simulink model "AcasXuClosedLoop_all_comp"  (Simulink 10.0)
settings: Solver=VariableStepAuto  StartTime=0.0  StopTime=20  RelTol=1e-3
libraries: neural x14040, simulink x2
(part 29 of 534)

== system AcasXuClosedLoop_all_comp/networks/Networks_m_7/AcasXu_1_7/Process Input 1 ==
  x : Inport  PortDimensions=5
      mask Display: e = 0.06;c1 = [120 230 180]/255;c2 = ([120 230 180]+0)/(256+128);c3 = ([120 230 180]+128)/(256+128);patch([0 1 1 0],[0 0 1 1],c1);patch([0 1 1 1-e 1-e 0+e],[0 0 1 1-e 0+e 0+e],c2);patch([0 0 1 1-e 0+e 0+e],[0 1 1 1-e 1-e 0+e],c3);plot([0 1 1 0 0],[0 0 1 1 0]);
  p : Outport  PortDimensions=5
      mask Display: e = 0.06;c1 = [120 230 180]/255;c2 = ([120 230 180]+0)/(256+128);c3 = ([120 230 180]+128)/(256+128);patch([0 1 1 0],[0 0 1 1],c1);patch([0 1 1 1-e 1-e 0+e],[0 0 1 1-e 0+e 0+e],c2);patch([0 0 1 1-e 0+e 0+e],[0 1 1 1-e 1-e 0+e],c3);plot([0 1 1 0 0],[0 0 1 1 0]);
  wires:
  x/1 -> p/1

== system AcasXuClosedLoop_all_comp/networks/Networks_m_7/AcasXu_1_7/Process Output 1 ==
  a : Inport  PortDimensions=5
      mask Display: e = 0.06;c1 = [120 230 180]/255;c2 = ([120 230 180]+0)/(256+128);c3 = ([120 230 180]+128)/(256+128);patch([0 1 1 0],[0 0 1 1],c1);patch([0 1 1 1-e 1-e 0+e],[0 0 1 1-e 0+e 0+e],c2);patch([0 0 1 1-e 0+e 0+e],[0 1 1 1-e 1-e 0+e],c3);plot([0 1 1 0 0],[0 0 1 1 0]);
  y : Outport  PortDimensions=5
      mask Display: e = 0.06;c1 = [120 230 180]/255;c2 = ([120 230 180]+0)/(256+128);c3 = ([120 230 180]+128)/(256+128);patch([0 1 1 0],[0 0 1 1],c1);patch([0 1 1 1-e 1-e 0+e],[0 0 1 1-e 0+e 0+e],c2);patch([0 0 1 1-e 0+e 0+e],[0 1 1 1-e 1-e 0+e],c3);plot([0 1 1 0 0],[0 0 1 1 0]);
  wires:
  a/1 -> y/1

== system AcasXuClosedLoop_all_comp/networks/Networks_m_7/AcasXu_2_7/Layer 1 ==
  "p{1}" : Inport  PortDimensions=5
      mask Display: e = 0.06;c1 = [120 230 180]/255;c2 = ([120 230 180]+0)/(256+128);c3 = ([120 230 180]+128)/(256+128);patch([0 1 1 0],[0 0 1 1],c1);patch([0 1 1 1-e 1-e 0+e],[0 0 1 1-e 0+e 0+e],c2);patch([0 0 1 1-e 0+e 0+e],[0 1 1 1-e 1-e 0+e],c3);plot([0 1 1 0 0],[0 0 1 1 0]);
  Delays 1 : Subsystem
      mask Display: e = 0.06;c1 = [120 150 230]/255;c2 = ([120 150 230]+0)/383;c3 = ([120 150 230]+128)/383;patch([0 1 1 0],[0 0 1 1],c1);patch([0 1 1 1-e 1-e 0+e],[0 0 1 1-e 0+e 0+e],c2);patch([0 0 1 1-e 0+e 0+e],[0 1 1 1-e 1-e 0+e],c3);plot([0 1 1 0 0],[0 0 1 1 0]);disp('0');
  "IW{1,1}" : Subsystem
      mask Display: e = 0.06;c1 = [120 150 230]/255;c2 = ([120 150 230]+0)/383;c3 = ([120 150 230]+128)/383;patch([0 1 1 0],[0 0 1 1],c1);patch([0 1 1 1-e 1-e 0+e],[0 0 1 1-e 0+e 0+e],c2);patch([0 0 1 1-e 0+e 0+e],[0 1 1 1-e 1-e 0+e],c3);plot([0 1 1 0 0],[0 0 1 1 0]);disp('W');
  "b{1}" : Constant  Value=[0.5234229999999999716209231337415985763072967529296875;0.11526999999999999746425061175614246167242527008056640625;-0.10370600000000000651123599482161807827651500701904296875;0.07139189999999999425295271748836967162787914276123046875;-0.1364549999999999929656269159750081598758697509765625;-0.48813600000000001433164698028122074902057647705078125;-0.257603000000000026403768060845322906970977783203125;-0.18697500000000000230926389122032560408115386962890625;-0.18462400000000001032418595059425570070743560791015625;0.068212400000000006361489113260176964104175567626953125;-0.2209480000000000055049298453013761900365352630615234375;-0.025648600000000000564970292771249660290777683258056640625;0.09991239999999999843449671743655926547944545745849609375;-0.0200602999999999996594279849659869796596467494964599609375;-0.5281029999999999891002744334400631487369537353515625;-0.01088579999999999929627403361109827528707683086395263671875;0.0243305000000000014315215679516768432222306728363037109375;-0.33044499999999998873789763820241205394268035888671875;0.12378599999999999325961397289574961178004741668701171875;0.0128616000000000008096634473986341618001461029052734375;-0.414652999999999993807620057850726880133152008056640625;-0.56115300000000001290345608140341937541961669921875;-0.007621389999999999588509158598981230170466005802154541015625;-0.751859000000000055052851166692562401294708251953125;-0.2264529999999999876347800409348565153777599334716796875;-0.003468859999999999825515129003861147793941199779510498046875;-0.32769700000000001605116040082066319882869720458984375;0.072733199999999997853450395268737338483333587646484375;-0.51464699999999996560262616185355000197887420654296875;0.01305259999999999927233762520017990027554333209991455078125;-0.5564270000000000049311665861750952899456024169921875;-0.29400199999999998556887703671236522495746612548828125;-0.457662999999999986489029879521694965660572052001953125;-0.1156689999999999940438755174909601919353008270263671875;-0.2459050000000000124611432283927570097148418426513671875;0.54282799999999997719868360945838503539562225341796875;-0.318711000000000022058799231672310270369052886962890625;-0.6888609999999999455866372954915277659893035888671875;-0.1474320000000000074891204349114559590816497802734375;-0.366167999999999993487875826758681796491146087646484375;0.1375860000000000138431488494461518712341785430908203125;-0.8581119999999999858886212678044103085994720458984375;0.0916486999999999996102673094355850480496883392333984375;0.08870020000000000681694700688240118324756622314453125;0.05061659999999999770547987054669647477567195892333984375;-0.376475999999999977330134015574003569781780242919921875;0.0620319000000000009276135415348107926547527313232421875;0.31844200000000000283506551568279974162578582763671875;-0.2102920000000000066986416413783445023000240325927734375;0.318415999999999976832754100541933439671993255615234375]
      mask Display: e = 0.06;c1 = [120 150 230]/255;c2 = ([120 150 230]+0)/383;c3 = ([120 150 230]+128)/383;patch([0 1 1 0],[0 0 1 1],c1);patch([0 1 1 1-e 1-e 0+e],[0 0 1 1-e 0+e 0+e],c2);patch([0 0 1 1-e 0+e 0+e],[0 1 1 1-e 1-e 0+e],c3);plot([0 1 1 0 0],[0 0 1 1 0]);disp('b');
  netsum : Sum  Inputs=2  SaturateOnIntegerOverflow=on  InputSameDT=on  OutDataTypeStr=Inherit: Same as first input
      mask Display: e = 0.06;c1 = [120 150 230]/255;c2 = ([120 150 230]+0)/383;c3 = ([120 150 230]+128)/383;patch([0 1 1 0],[0 0 1 1],c1);patch([0 1 1 1-e 1-e 0+e],[0 0 1 1-e 0+e 0+e],c2);patch([0 0 1 1-e 0+e 0+e],[0 1 1 1-e 1-e 0+e],c3);plot([0 1 1 0 0],[0 0 1 1 0]);disp('+');
  poslin : neural/Transfer Functions/poslin
      settings: SourceProductName=Deep Learning Toolbox  SourceProductBaseCode=NN
  "a{1}" : Outport  InitialOutput=[0;0;0;0;0;0;0;0;0;0;0;0;0;0;0;0;0;0;0;0;0;0;0;0;0;0;0;0;0;0;0;0;0;0;0;0;0;0;0;0;0;0;0;0;0;0;0;0;0;0]
      mask Display: e = 0.06;c1 = [120 230 180]/255;c2 = ([120 230 180]+0)/(256+128);c3 = ([120 230 180]+128)/(256+128);patch([0 1 1 0],[0 0 1 1],c1);patch([0 1 1 1-e 1-e 0+e],[0 0 1 1-e 0+e 0+e],c2);patch([0 0 1 1-e 0+e 0+e],[0 1 1 1-e 1-e 0+e],c3);plot([0 1 1 0 0],[0 0 1 1 0]);
  wires:
  poslin/1 -> "a{1}"/1
  netsum/1 -> poslin/1
  "b{1}"/1 -> netsum/2
  "IW{1,1}"/1("iz{1,1}") -> netsum/1
  Delays 1/1("pd{1,1}") -> "IW{1,1}"/1("pd{1,1}")
  "p{1}"/1 -> Delays 1/1("p{1}")

== system AcasXuClosedLoop_all_comp/networks/Networks_m_7/AcasXu_2_7/Layer 2 ==
  "a{1} " : Inport  PortDimensions=50
      mask Display: e = 0.06;c1 = [120 230 180]/255;c2 = ([120 230 180]+0)/(256+128);c3 = ([120 230 180]+128)/(256+128);patch([0 1 1 0],[0 0 1 1],c1);patch([0 1 1 1-e 1-e 0+e],[0 0 1 1-e 0+e 0+e],c2);patch([0 0 1 1-e 0+e 0+e],[0 1 1 1-e 1-e 0+e],c3);plot([0 1 1 0 0],[0 0 1 1 0]);
  Delays 1 : Subsystem
      mask Display: e = 0.06;c1 = [120 150 230]/255;c2 = ([120 150 230]+0)/383;c3 = ([120 150 230]+128)/383;patch([0 1 1 0],[0 0 1 1],c1);patch([0 1 1 1-e 1-e 0+e],[0 0 1 1-e 0+e 0+e],c2);patch([0 0 1 1-e 0+e 0+e],[0 1 1 1-e 1-e 0+e],c3);plot([0 1 1 0 0],[0 0 1 1 0]);disp('0');
  "LW{2,1}" : Subsystem
      mask Display: e = 0.06;c1 = [120 150 230]/255;c2 = ([120 150 230]+0)/383;c3 = ([120 150 230]+128)/383;patch([0 1 1 0],[0 0 1 1],c1);patch([0 1 1 1-e 1-e 0+e],[0 0 1 1-e 0+e 0+e],c2);patch([0 0 1 1-e 0+e 0+e],[0 1 1 1-e 1-e 0+e],c3);plot([0 1 1 0 0],[0 0 1 1 0]);disp('W');
  "b{2}" : Constant  Value=[-0.304109000000000018193446749137365259230136871337890625;-0.8032500000000000195399252334027551114559173583984375;0.790129999999999999005240169935859739780426025390625;-0.92732599999999998363620079544489271938800811767578125;-1.424350000000000004973799150320701301097869873046875;-0.0396675999999999973066877601013402454555034637451171875;-0.015490600000000000202593497533598565496504306793212890625;-0.25707000000000002071232074740692041814327239990234375;-0.63598900000000002652456032592453993856906890869140625;0.2584779999999999855475607546395622193813323974609375;-1.148800000000000043343106881366111338138580322265625;0.802705000000000001847411112976260483264923095703125;-0.306186000000000013709922086491133086383342742919921875;-1.356139999999999901092451182194054126739501953125;0.231009999999999993125499031521030701696872711181640625;-0.3563169999999999948414597383816726505756378173828125;-0.0256099000000000014132250925058542634360492229461669921875;-1.553020000000000067075234255753457546234130859375;-0.016525800000000000211652917414539842866361141204833984375;0.03723400000000000320543591669775196351110935211181640625;1.204029999999999933635308480006642639636993408203125;0.884560000000000012931877790833823382854461669921875;0.7280889999999999862012600715388543903827667236328125;-0.028294199999999998518251942414281074889004230499267578125;-1.2106399999999999383959448095993138849735260009765625;0.61121700000000001029576424116385169327259063720703125;-0.01231570000000000060513816180218782392330467700958251953125;0.130025000000000001687538997430237941443920135498046875;-0.00610049000000000007926104217403917573392391204833984375;0.78768800000000005478995035446132533252239227294921875;0.9423880000000000034532376957940869033336639404296875;-0.0214050999999999998213429108773198095150291919708251953125;-0.92050200000000004241229589752038009464740753173828125;-0.0735737999999999947586815096656209789216518402099609375;0.03393319999999999669881844965857453644275665283203125;0.382917000000000007364775456153438426554203033447265625;-1.018219999999999902939862295170314610004425048828125;-0.015413400000000000711342096337830298580229282379150390625;0.5833850000000000424194013248779810965061187744140625;-0.0159619000000000010874856570808333344757556915283203125;0.58658299999999996554578274299274198710918426513671875;0.65938499999999999889865875957184471189975738525390625;0.59477700000000000013500311979441903531551361083984375;1.089960000000000039932501749717630445957183837890625;-0.0159193000000000008109513061071993433870375156402587890625;-0.4555919999999999969730879456619732081890106201171875;-0.109399999999999997246646898929611779749393463134765625;0.57275500000000001410427330483798868954181671142578125;-0.0150588000000000006572964394990776781924068927764892578125;-0.0170725000000000008693046282814975711517035961151123046875]
      mask Display: e = 0.06;c1 = [120 150 230]/255;c2 = ([120 150 230]+0)/383;c3 = ([120 150 230]+128)/383;patch([0 1 1 0],[0 0 1 1],c1);patch([0 1 1 1-e 1-e 0+e],[0 0 1 1-e 0+e 0+e],c2);patch([0 0 1 1-e 0+e 0+e],[0 1 1 1-e 1-e 0+e],c3);plot([0 1 1 0 0],[0 0 1 1 0]);disp('b');
  netsum : Sum  Inputs=2  SaturateOnIntegerOverflow=on  InputSameDT=on  OutDataTypeStr=Inherit: Same as first input
      mask Display: e = 0.06;c1 = [120 150 230]/255;c2 = ([120 150 230]+0)/383;c3 = ([120 150 230]+128)/383;patch([0 1 1 0],[0 0 1 1],c1);patch([0 1 1 1-e 1-e 0+e],[0 0 1 1-e 0+e 0+e],c2);patch([0 0 1 1-e 0+e 0+e],[0 1 1 1-e 1-e 0+e],c3);plot([0 1 1 0 0],[0 0 1 1 0]);disp('+');
  poslin : neural/Transfer Functions/poslin
      settings: SourceProductName=Deep Learning Toolbox  SourceProductBaseCode=NN
  "a{2}" : Outport  InitialOutput=[0;0;0;0;0;0;0;0;0;0;0;0;0;0;0;0;0;0;0;0;0;0;0;0;0;0;0;0;0;0;0;0;0;0;0;0;0;0;0;0;0;0;0;0;0;0;0;0;0;0]
      mask Display: e = 0.06;c1 = [120 230 180]/255;c2 = ([120 230 180]+0)/(256+128);c3 = ([120 230 180]+128)/(256+128);patch([0 1 1 0],[0 0 1 1],c1);patch([0 1 1 1-e 1-e 0+e],[0 0 1 1-e 0+e 0+e],c2);patch([0 0 1 1-e 0+e 0+e],[0 1 1 1-e 1-e 0+e],c3);plot([0 1 1 0 0],[0 0 1 1 0]);
  wires:
  poslin/1 -> "a{2}"/1
  netsum/1 -> poslin/1
  "b{2}"/1 -> netsum/2
  "LW{2,1}"/1("lz{2,1}") -> netsum/1
  Delays 1/1("pd{2,1}") -> "LW{2,1}"/1("ad{2,1}")
  "a{1} "/1 -> Delays 1/1("p{2}")

== system AcasXuClosedLoop_all_comp/networks/Networks_m_7/AcasXu_2_7/Layer 3 ==
  "a{2} " : Inport  PortDimensions=50
      mask Display: e = 0.06;c1 = [120 230 180]/255;c2 = ([120 230 180]+0)/(256+128);c3 = ([120 230 180]+128)/(256+128);patch([0 1 1 0],[0 0 1 1],c1);patch([0 1 1 1-e 1-e 0+e],[0 0 1 1-e 0+e 0+e],c2);patch([0 0 1 1-e 0+e 0+e],[0 1 1 1-e 1-e 0+e],c3);plot([0 1 1 0 0],[0 0 1 1 0]);
  Delays 1 : Subsystem
      mask Display: e = 0.06;c1 = [120 150 230]/255;c2 = ([120 150 230]+0)/383;c3 = ([120 150 230]+128)/383;patch([0 1 1 0],[0 0 1 1],c1);patch([0 1 1 1-e 1-e 0+e],[0 0 1 1-e 0+e 0+e],c2);patch([0 0 1 1-e 0+e 0+e],[0 1 1 1-e 1-e 0+e],c3);plot([0 1 1 0 0],[0 0 1 1 0]);disp('0');
  "LW{3,2}" : Subsystem
      mask Display: e = 0.06;c1 = [120 150 230]/255;c2 = ([120 150 230]+0)/383;c3 = ([120 150 230]+128)/383;patch([0 1 1 0],[0 0 1 1],c1);patch([0 1 1 1-e 1-e 0+e],[0 0 1 1-e 0+e 0+e],c2);patch([0 0 1 1-e 0+e 0+e],[0 1 1 1-e 1-e 0+e],c3);plot([0 1 1 0 0],[0 0 1 1 0]);disp('W');
  "b{3}" : Constant  Value=[-0.05460299999999999875921474767892505042254924774169921875;-1.51025000000000009237055564881302416324615478515625;-1.2421100000000000473932004751986823976039886474609375;-1.3791700000000000070343730840249918401241302490234375;-2.25302999999999986613374858279712498188018798828125;-0.06695610000000000450182113809205475263297557830810546875;0.034173500000000002485567307530800462700426578521728515625;0.193807000000000007045031225061393342912197113037109375;-3.100969999999999782147597215953283011913299560546875;-0.327037999999999995370814076522947289049625396728515625;-0.93910800000000005383071766118519008159637451171875;-0.40935500000000002440714297335944138467311859130859375;-1.8680499999999999882760448599583469331264495849609375;0.89845900000000000762412355470587499439716339111328125;-0.33551500000000000767386154620908200740814208984375;-0.1792789999999999939195305387329426594078540802001953125;0.65444899999999994744115383582538925111293792724609375;0.42373899999999997678656882271752692759037017822265625;0.55749300000000001631406121305190026760101318359375;-0.1454959999999999864517263858942897059023380279541015625;0.63791100000000000580513415116001851856708526611328125;0.41257800000000000029132252166164107620716094970703125;0.252634000000000025210056264768354594707489013671875;0.84931199999999995586819068194017745554447174072265625;-0.51852200000000003843325657726381905376911163330078125;0.1806649999999999922639659644119092263281345367431640625;-0.476061000000000011933565247090882621705532073974609375;0.197778000000000009350742402602918446063995361328125;-2.60480000000000000426325641456060111522674560546875;0.3153580000000000271853650701814331114292144775390625;-1.600410000000000110276232589967548847198486328125;0.336000000000000020872192862952942959964275360107421875;-2.201700000000000212452277992269955575466156005859375;0.0999147999999999980591525172712863422930240631103515625;-2.7599900000000001654143488849513232707977294921875;-0.0243119000000000008709921672789278090931475162506103515625;0.65167699999999995075228298446745611727237701416015625;0.319890999999999980918374831162509508430957794189453125;2.00330999999999992411403582082130014896392822265625;-0.9242879999999999984794385454733856022357940673828125;-1.597140000000000004121147867408581078052520751953125;0.5246269999999999544826323472079820930957794189453125;-1.27812999999999998834709913353435695171356201171875;-3.459799999999999986499688020558096468448638916015625;0.37772200000000000219557705349870957434177398681640625;0.95909100000000002683719912965898402035236358642578125;-2.129820000000000046469494918710552155971527099609375;0.08330690000000000317026405127762700431048870086669921875;-0.83285500000000001197264509755768813192844390869140625;-0.5695580000000000087112539404188282787799835205078125]
      mask Display: e = 0.06;c1 = [120 150 230]/255;c2 = ([120 150 230]+0)/383;c3 = ([120 150 230]+128)/383;patch([0 1 1 0],[0 0 1 1],c1);patch([0 1 1 1-e 1-e 0+e],[0 0 1 1-e 0+e 0+e],c2);patch([0 0 1 1-e 0+e 0+e],[0 1 1 1-e 1-e 0+e],c3);plot([0 1 1 0 0],[0 0 1 1 0]);disp('b');
  netsum : Sum  Inputs=2  SaturateOnIntegerOverflow=on  InputSameDT=on  OutDataTypeStr=Inherit: Same as first input
      mask Display: e = 0.06;c1 = [120 150 230]/255;c2 = ([120 150 230]+0)/383;c3 = ([120 150 230]+128)/383;patch([0 1 1 0],[0 0 1 1],c1);patch([0 1 1 1-e 1-e 0+e],[0 0 1 1-e 0+e 0+e],c2);patch([0 0 1 1-e 0+e 0+e],[0 1 1 1-e 1-e 0+e],c3);plot([0 1 1 0 0],[0 0 1 1 0]);disp('+');
  poslin : neural/Transfer Functions/poslin
      settings: SourceProductName=Deep Learning Toolbox  SourceProductBaseCode=NN
  "a{3}" : Outport  InitialOutput=[0;0;0;0;0;0;0;0;0;0;0;0;0;0;0;0;0;0;0;0;0;0;0;0;0;0;0;0;0;0;0;0;0;0;0;0;0;0;0;0;0;0;0;0;0;0;0;0;0;0]
      mask Display: e = 0.06;c1 = [120 230 180]/255;c2 = ([120 230 180]+0)/(256+128);c3 = ([120 230 180]+128)/(256+128);patch([0 1 1 0],[0 0 1 1],c1);patch([0 1 1 1-e 1-e 0+e],[0 0 1 1-e 0+e 0+e],c2);patch([0 0 1 1-e 0+e 0+e],[0 1 1 1-e 1-e 0+e],c3);plot([0 1 1 0 0],[0 0 1 1 0]);
  wires:
  poslin/1 -> "a{3}"/1
  netsum/1 -> poslin/1
  "b{3}"/1 -> netsum/2
  "LW{3,2}"/1("lz{3,2}") -> netsum/1
  Delays 1/1("pd{3,2}") -> "LW{3,2}"/1("ad{3,2}")
  "a{2} "/1 -> Delays 1/1("p{3}")

== system AcasXuClosedLoop_all_comp/networks/Networks_m_7/AcasXu_2_7/Layer 4 ==
  "a{3} " : Inport  PortDimensions=50
      mask Display: e = 0.06;c1 = [120 230 180]/255;c2 = ([120 230 180]+0)/(256+128);c3 = ([120 230 180]+128)/(256+128);patch([0 1 1 0],[0 0 1 1],c1);patch([0 1 1 1-e 1-e 0+e],[0 0 1 1-e 0+e 0+e],c2);patch([0 0 1 1-e 0+e 0+e],[0 1 1 1-e 1-e 0+e],c3);plot([0 1 1 0 0],[0 0 1 1 0]);
  Delays 1 : Subsystem
      mask Display: e = 0.06;c1 = [120 150 230]/255;c2 = ([120 150 230]+0)/383;c3 = ([120 150 230]+128)/383;patch([0 1 1 0],[0 0 1 1],c1);patch([0 1 1 1-e 1-e 0+e],[0 0 1 1-e 0+e 0+e],c2);patch([0 0 1 1-e 0+e 0+e],[0 1 1 1-e 1-e 0+e],c3);plot([0 1 1 0 0],[0 0 1 1 0]);disp('0');
  "LW{4,3}" : Subsystem
      mask Display: e = 0.06;c1 = [120 150 230]/255;c2 = ([120 150 230]+0)/383;c3 = ([120 150 230]+128)/383;patch([0 1 1 0],[0 0 1 1],c1);patch([0 1 1 1-e 1-e 0+e],[0 0 1 1-e 0+e 0+e],c2);patch([0 0 1 1-e 0+e 0+e],[0 1 1 1-e 1-e 0+e],c3);plot([0 1 1 0 0],[0 0 1 1 0]);disp('W');
  "b{4}" : Constant  Value=[-3.171460000000000167830194186535663902759552001953125;0.58932200000000001249844672202016226947307586669921875;-1.4313000000000000166977542903623543679714202880859375;-0.00948605999999999928429250672934358590282499790191650390625;-1.1083700000000000773781039242749102413654327392578125;-1.734809999999999963193886287626810371875762939453125;0.2420529999999999903881331420052447356283664703369140625;0.52557299999999995687716136671951971948146820068359375;-1.92884000000000010999201549566350877285003662109375;2.644420000000000214868123293854296207427978515625;-1.62112999999999995992538970313034951686859130859375;-0.306956999999999979866771582237561233341693878173828125;-4.1185700000000000642330633127130568027496337890625;-1.148260000000000058406612879480235278606414794921875;3.008919999999999816964191268198192119598388671875;2.865229999999999943582906780648045241832733154296875;0.259365999999999985448084771633148193359375;0.4819539999999999935198502498678863048553466796875;0.529708999999999985419663062202744185924530029296875;0.65275499999999997413624441833235323429107666015625;-1.504350000000000076028072726330719888210296630859375;0.90964400000000000812150346973794512450695037841796875;2.1580799999999999982946974341757595539093017578125;3.21356999999999981554310579667799174785614013671875;-0.869021000000000043428372009657323360443115234375;0.75575099999999995059596358260023407638072967529296875;3.0660199999999999675992512493394315242767333984375;-1.489640000000000075175421443418599665164947509765625;0.9238079999999999625259761160123161971569061279296875;1.9984900000000000996891458271420560777187347412109375;0.30948900000000001409006245012278668582439422607421875;-1.6852400000000000712674363967380486428737640380859375;-1.0825400000000000577671244172961451113224029541015625;1.5658000000000000806466005087713710963726043701171875;0.288138999999999978474107820147764869034290313720703125;-0.67373899999999997678656882271752692759037017822265625;-2.964230000000000142534872793476097285747528076171875;-3.2730399999999999494093572138808667659759521484375;0.484302999999999983504750389329274185001850128173828125;0.44874300000000000299138491755002178251743316650390625;-1.372409999999999907771552898338995873928070068359375;1.1985099999999999642597003912669606506824493408203125;-0.0258034000000000006413980457864454365335404872894287109375;-2.59421999999999997044142219237983226776123046875;-0.49597999999999997644550830955267883837223052978515625;-0.75792599999999998860999994576559402048587799072265625;-0.99425799999999997513810967575409449636936187744140625;-1.7488300000000001066524646375910378992557525634765625;-0.1426099999999999867750233306651352904736995697021484375;-1.2576799999999999091215840962831862270832061767578125]
      mask Display: e = 0.06;c1 = [120 150 230]/255;c2 = ([120 150 230]+0)/383;c3 = ([120 150 230]+128)/383;patch([0 1 1 0],[0 0 1 1],c1);patch([0 1 1 1-e 1-e 0+e],[0 0 1 1-e 0+e 0+e],c2);patch([0 0 1 1-e 0+e 0+e],[0 1 1 1-e 1-e 0+e],c3);plot([0 1 1 0 0],[0 0 1 1 0]);disp('b');
  netsum : Sum  Inputs=2  SaturateOnIntegerOverflow=on  InputSameDT=on  OutDataTypeStr=Inherit: Same as first input
      mask Display: e = 0.06;c1 = [120 150 230]/255;c2 = ([120 150 230]+0)/383;c3 = ([120 150 230]+128)/383;patch([0 1 1 0],[0 0 1 1],c1);patch([0 1 1 1-e 1-e 0+e],[0 0 1 1-e 0+e 0+e],c2);patch([0 0 1 1-e 0+e 0+e],[0 1 1 1-e 1-e 0+e],c3);plot([0 1 1 0 0],[0 0 1 1 0]);disp('+');
  poslin : neural/Transfer Functions/poslin
      settings: SourceProductName=Deep Learning Toolbox  SourceProductBaseCode=NN
  "a{4}" : Outport  InitialOutput=[0;0;0;0;0;0;0;0;0;0;0;0;0;0;0;0;0;0;0;0;0;0;0;0;0;0;0;0;0;0;0;0;0;0;0;0;0;0;0;0;0;0;0;0;0;0;0;0;0;0]
      mask Display: e = 0.06;c1 = [120 230 180]/255;c2 = ([120 230 180]+0)/(256+128);c3 = ([120 230 180]+128)/(256+128);patch([0 1 1 0],[0 0 1 1],c1);patch([0 1 1 1-e 1-e 0+e],[0 0 1 1-e 0+e 0+e],c2);patch([0 0 1 1-e 0+e 0+e],[0 1 1 1-e 1-e 0+e],c3);plot([0 1 1 0 0],[0 0 1 1 0]);
  wires:
  poslin/1 -> "a{4}"/1
  netsum/1 -> poslin/1
  "b{4}"/1 -> netsum/2
  "LW{4,3}"/1("lz{4,3}") -> netsum/1
  Delays 1/1("pd{4,3}") -> "LW{4,3}"/1("ad{4,3}")
  "a{3} "/1 -> Delays 1/1("p{4}")

== system AcasXuClosedLoop_all_comp/networks/Networks_m_7/AcasXu_2_7/Layer 5 ==
  "a{4} " : Inport  PortDimensions=50
      mask Display: e = 0.06;c1 = [120 230 180]/255;c2 = ([120 230 180]+0)/(256+128);c3 = ([120 230 180]+128)/(256+128);patch([0 1 1 0],[0 0 1 1],c1);patch([0 1 1 1-e 1-e 0+e],[0 0 1 1-e 0+e 0+e],c2);patch([0 0 1 1-e 0+e 0+e],[0 1 1 1-e 1-e 0+e],c3);plot([0 1 1 0 0],[0 0 1 1 0]);
  Delays 1 : Subsystem
      mask Display: e = 0.06;c1 = [120 150 230]/255;c2 = ([120 150 230]+0)/383;c3 = ([120 150 230]+128)/383;patch([0 1 1 0],[0 0 1 1],c1);patch([0 1 1 1-e 1-e 0+e],[0 0 1 1-e 0+e 0+e],c2);patch([0 0 1 1-e 0+e 0+e],[0 1 1 1-e 1-e 0+e],c3);plot([0 1 1 0 0],[0 0 1 1 0]);disp('0');
  "LW{5,4}" : Subsystem
      mask Display: e = 0.06;c1 = [120 150 230]/255;c2 = ([120 150 230]+0)/383;c3 = ([120 150 230]+128)/383;patch([0 1 1 0],[0 0 1 1],c1);patch([0 1 1 1-e 1-e 0+e],[0 0 1 1-e 0+e 0+e],c2);patch([0 0 1 1-e 0+e 0+e],[0 1 1 1-e 1-e 0+e],c3);plot([0 1 1 0 0],[0 0 1 1 0]);disp('W');
  "b{5}" : Constant  Value=[-2.079520000000000035100811146548949182033538818359375;-0.7507509999999999461550714840996079146862030029296875;-5.86850999999999967116082189022563397884368896484375;-2.434839999999999893276481088832952082157135009765625;-1.22506000000000003780087354243732988834381103515625;-0.728685000000000027142732506035827100276947021484375;1.1832300000000000039790393202565610408782958984375;1.4805099999999999926814098216709680855274200439453125;-3.37661999999999995480948200565762817859649658203125;-4.427550000000000096633812063373625278472900390625;-0.91487200000000001853805997598101384937763214111328125;-0.990815000000000001278976924368180334568023681640625;-2.118180000000000173798753166920505464076995849609375;-1.6213100000000000289190893454360775649547576904296875;-3.217779999999999862581034903996624052524566650390625;-1.886060000000000069775296651641838252544403076171875;1.42964000000000002188471626141108572483062744140625;-1.4949399999999999355537738665589131414890289306640625;-2.1922899999999998499333742074668407440185546875;-0.930336000000000051812776291626505553722381591796875;-1.27583000000000001961097950697876513004302978515625;0.353312000000000014932055591998505406081676483154296875;-0.2018459999999999976427744741158676333725452423095703125;-1.907340000000000035385028240852989256381988525390625;-1.4374700000000000255084842137875966727733612060546875;-0.2414920000000000122053478435191209428012371063232421875;-3.781140000000000167545977092231623828411102294921875;1.17982999999999993434585121576674282550811767578125;-1.036189999999999944435558063560165464878082275390625;0.147301999999999988499865821722778491675853729248046875;-0.06721950000000000147171164144310750998556613922119140625;0.25942399999999998794208977415109984576702117919921875;-3.31106999999999995765165294869802892208099365234375;-1.4908500000000000085265128291212022304534912109375;2.671730000000000160298441187478601932525634765625;-1.1877299999999999524646909776492975652217864990234375;-2.4264700000000001267608240596018731594085693359375;0.7867859999999999853770304980571381747722625732421875;0.0411289999999999988933296890536439605057239532470703125;-0.56640900000000005132250180395203642547130584716796875;-0.73486700000000004795452923644916154444217681884765625;2.414759999999999795505800648243166506290435791015625;-1.7295400000000000773070496506989002227783203125;-0.10725200000000000011279865930191590450704097747802734375;0.8372749999999999914734871708787977695465087890625;-0.56637499999999996180832795289461500942707061767578125;-0.68165500000000001090683099391753785312175750732421875;-2.791110000000000201936245503020472824573516845703125;-0.76091600000000003678479743030038662254810333251953125;-0.835536999999999974164666127762757241725921630859375]
      mask Display: e = 0.06;c1 = [120 150 230]/255;c2 = ([120 150 230]+0)/383;c3 = ([120 150 230]+128)/383;patch([0 1 1 0],[0 0 1 1],c1);patch([0 1 1 1-e 1-e 0+e],[0 0 1 1-e 0+e 0+e],c2);patch([0 0 1 1-e 0+e 0+e],[0 1 1 1-e 1-e 0+e],c3);plot([0 1 1 0 0],[0 0 1 1 0]);disp('b');
  netsum : Sum  Inputs=2  SaturateOnIntegerOverflow=on  InputSameDT=on  OutDataTypeStr=Inherit: Same as first input
      mask Display: e = 0.06;c1 = [120 150 230]/255;c2 = ([120 150 230]+0)/383;c3 = ([120 150 230]+128)/383;patch([0 1 1 0],[0 0 1 1],c1);patch([0 1 1 1-e 1-e 0+e],[0 0 1 1-e 0+e 0+e],c2);patch([0 0 1 1-e 0+e 0+e],[0 1 1 1-e 1-e 0+e],c3);plot([0 1 1 0 0],[0 0 1 1 0]);disp('+');
  poslin : neural/Transfer Functions/poslin
      settings: SourceProductName=Deep Learning Toolbox  SourceProductBaseCode=NN
  "a{5}" : Outport  InitialOutput=[0;0;0;0;0;0;0;0;0;0;0;0;0;0;0;0;0;0;0;0;0;0;0;0;0;0;0;0;0;0;0;0;0;0;0;0;0;0;0;0;0;0;0;0;0;0;0;0;0;0]
      mask Display: e = 0.06;c1 = [120 230 180]/255;c2 = ([120 230 180]+0)/(256+128);c3 = ([120 230 180]+128)/(256+128);patch([0 1 1 0],[0 0 1 1],c1);patch([0 1 1 1-e 1-e 0+e],[0 0 1 1-e 0+e 0+e],c2);patch([0 0 1 1-e 0+e 0+e],[0 1 1 1-e 1-e 0+e],c3);plot([0 1 1 0 0],[0 0 1 1 0]);
  wires:
  poslin/1 -> "a{5}"/1
  netsum/1 -> poslin/1
  "b{5}"/1 -> netsum/2
  "LW{5,4}"/1("lz{5,4}") -> netsum/1
  Delays 1/1("pd{5,4}") -> "LW{5,4}"/1("ad{5,4}")
  "a{4} "/1 -> Delays 1/1("p{5}")

== system AcasXuClosedLoop_all_comp/networks/Networks_m_7/AcasXu_2_7/Layer 6 ==
  "a{5} " : Inport  PortDimensions=50
      mask Display: e = 0.06;c1 = [120 230 180]/255;c2 = ([120 230 180]+0)/(256+128);c3 = ([120 230 180]+128)/(256+128);patch([0 1 1 0],[0 0 1 1],c1);patch([0 1 1 1-e 1-e 0+e],[0 0 1 1-e 0+e 0+e],c2);patch([0 0 1 1-e 0+e 0+e],[0 1 1 1-e 1-e 0+e],c3);plot([0 1 1 0 0],[0 0 1 1 0]);
  Delays 1 : Subsystem
      mask Display: e = 0.06;c1 = [120 150 230]/255;c2 = ([120 150 230]+0)/383;c3 = ([120 150 230]+128)/383;patch([0 1 1 0],[0 0 1 1],c1);patch([0 1 1 1-e 1-e 0+e],[0 0 1 1-e 0+e 0+e],c2);patch([0 0 1 1-e 0+e 0+e],[0 1 1 1-e 1-e 0+e],c3);plot([0 1 1 0 0],[0 0 1 1 0]);disp('0');
  "LW{6,5}" : Subsystem
      mask Display: e = 0.06;c1 = [120 150 230]/255;c2 = ([120 150 230]+0)/383;c3 = ([120 150 230]+128)/383;patch([0 1 1 0],[0 0 1 1],c1);patch([0 1 1 1-e 1-e 0+e],[0 0 1 1-e 0+e 0+e],c2);patch([0 0 1 1-e 0+e 0+e],[0 1 1 1-e 1-e 0+e],c3);plot([0 1 1 0 0],[0 0 1 1 0]);disp('W');
  "b{6}" : Constant  Value=[0.045646499999999999575006626173490076325833797454833984375;-1.1119499999999999939603867460391484200954437255859375;1.2160200000000001008260142043582163751125335693359375;-0.500237999999999960465402182308025658130645751953125;-0.25165300000000001556799134050379507243633270263671875;-0.95177800000000001290345608140341937541961669921875;5.68015000000000025437429940211586654186248779296875;-0.480746999999999979902298719025566242635250091552734375;-7.28071000000000001506350599811412394046783447265625;0.271019999999999983142373594091623090207576751708984375;-3.054679999999999839843667359673418104648590087890625;1.1532400000000000428457269663340412080287933349609375;3.02245000000000008100187187665142118930816650390625;3.494520000000000070627947934553958475589752197265625;-5.82957999999999998408384271897375583648681640625;0.410248000000000001552535877635818906128406524658203125;-5.28859000000000012420287021086551249027252197265625;3.97243000000000012761347534251399338245391845703125;2.45551999999999992496668710373342037200927734375;0.206361999999999989885424156454973854124546051025390625;-1.8719500000000000028421709430404007434844970703125;-5.3410700000000002063416104647330939769744873046875;-6.03371000000000012875034371973015367984771728515625;-5.09347999999999956344254314899444580078125;-2.43236000000000007759126674500294029712677001953125;-5.70167000000000001591615728102624416351318359375;-6.84029000000000042547299017314799129962921142578125;-5.0742799999999999016608853708021342754364013671875;1.1467899999999999760547098048846237361431121826171875;-0.7053190000000000292601498586009256541728973388671875;-1.787570000000000103312913779518567025661468505859375;-9.2444199999999998595967554138042032718658447265625;-5.9402699999999999391775418189354240894317626953125;-5.53108000000000021856294551980681717395782470703125;3.2964999999999999857891452847979962825775146484375;3.86348000000000002529532139305956661701202392578125;1.4553799999999998959765434847213327884674072265625;-6.7179900000000003501554601825773715972900390625;-2.670949999999999935340611045830883085727691650390625;-0.1906090000000000006519229600598919205367565155029296875;0.134909000000000001140421090894960798323154449462890625;-2.3918400000000001881517164292745292186737060546875;1.7484200000000000851940740176360122859477996826171875;0.5655360000000000386677356800646521151065826416015625;-8.502150000000000318323145620524883270263671875;1.414309999999999956088458930025808513164520263671875;0.70750199999999996425259496390935964882373809814453125;-1.9529300000000000547828449271037243306636810302734375;1.6271400000000000307665004584123380482196807861328125;-0.0887532000000000043105075064886477775871753692626953125]
      mask Display: e = 0.06;c1 = [120 150 230]/255;c2 = ([120 150 230]+0)/383;c3 = ([120 150 230]+128)/383;patch([0 1 1 0],[0 0 1 1],c1);patch([0 1 1 1-e 1-e 0+e],[0 0 1 1-e 0+e 0+e],c2);patch([0 0 1 1-e 0+e 0+e],[0 1 1 1-e 1-e 0+e],c3);plot([0 1 1 0 0],[0 0 1 1 0]);disp('b');
  netsum : Sum  Inputs=2  SaturateOnIntegerOverflow=on  InputSameDT=on  OutDataTypeStr=Inherit: Same as first input
      mask Display: e = 0.06;c1 = [120 150 230]/255;c2 = ([120 150 230]+0)/383;c3 = ([120 150 230]+128)/383;patch([0 1 1 0],[0 0 1 1],c1);patch([0 1 1 1-e 1-e 0+e],[0 0 1 1-e 0+e 0+e],c2);patch([0 0 1 1-e 0+e 0+e],[0 1 1 1-e 1-e 0+e],c3);plot([0 1 1 0 0],[0 0 1 1 0]);disp('+');
  poslin : neural/Transfer Functions/poslin
      settings: SourceProductName=Deep Learning Toolbox  SourceProductBaseCode=NN
  "a{6}" : Outport  InitialOutput=[0;0;0;0;0;0;0;0;0;0;0;0;0;0;0;0;0;0;0;0;0;0;0;0;0;0;0;0;0;0;0;0;0;0;0;0;0;0;0;0;0;0;0;0;0;0;0;0;0;0]
      mask Display: e = 0.06;c1 = [120 230 180]/255;c2 = ([120 230 180]+0)/(256+128);c3 = ([120 230 180]+128)/(256+128);patch([0 1 1 0],[0 0 1 1],c1);patch([0 1 1 1-e 1-e 0+e],[0 0 1 1-e 0+e 0+e],c2);patch([0 0 1 1-e 0+e 0+e],[0 1 1 1-e 1-e 0+e],c3);plot([0 1 1 0 0],[0 0 1 1 0]);
  wires:
  poslin/1 -> "a{6}"/1
  netsum/1 -> poslin/1
  "b{6}"/1 -> netsum/2
  "LW{6,5}"/1("lz{6,5}") -> netsum/1
  Delays 1/1("pd{6,5}") -> "LW{6,5}"/1("ad{6,5}")
  "a{5} "/1 -> Delays 1/1("p{6}")

== system AcasXuClosedLoop_all_comp/networks/Networks_m_7/AcasXu_2_7/Layer 7 ==
  "a{6} " : Inport  PortDimensions=50
      mask Display: e = 0.06;c1 = [120 230 180]/255;c2 = ([120 230 180]+0)/(256+128);c3 = ([120 230 180]+128)/(256+128);patch([0 1 1 0],[0 0 1 1],c1);patch([0 1 1 1-e 1-e 0+e],[0 0 1 1-e 0+e 0+e],c2);patch([0 0 1 1-e 0+e 0+e],[0 1 1 1-e 1-e 0+e],c3);plot([0 1 1 0 0],[0 0 1 1 0]);
  Delays 1 : Subsystem
      mask Display: e = 0.06;c1 = [120 150 230]/255;c2 = ([120 150 230]+0)/383;c3 = ([120 150 230]+128)/383;patch([0 1 1 0],[0 0 1 1],c1);patch([0 1 1 1-e 1-e 0+e],[0 0 1 1-e 0+e 0+e],c2);patch([0 0 1 1-e 0+e 0+e],[0 1 1 1-e 1-e 0+e],c3);plot([0 1 1 0 0],[0 0 1 1 0]);disp('0');
  "LW{7,6}" : Subsystem
      mask Display: e = 0.06;c1 = [120 150 230]/255;c2 = ([120 150 230]+0)/383;c3 = ([120 150 230]+128)/383;patch([0 1 1 0],[0 0 1 1],c1);patch([0 1 1 1-e 1-e 0+e],[0 0 1 1-e 0+e 0+e],c2);patch([0 0 1 1-e 0+e 0+e],[0 1 1 1-e 1-e 0+e],c3);plot([0 1 1 0 0],[0 0 1 1 0]);disp('W');
  "b{7}" : Constant  Value=[-0.0201921000000000010199396882626388105563819408416748046875;-0.0197996999999999999386712801197063527069985866546630859375;0.018020899999999999419042495674148085527122020721435546875;-0.0186712999999999983147258575399973778985440731048583984375;0.01821330000000000171045400065850117243826389312744140625]
      mask Display: e = 0.06;c1 = [120 150 230]/255;c2 = ([120 150 230]+0)/383;c3 = ([120 150 230]+128)/383;patch([0 1 1 0],[0 0 1 1],c1);patch([0 1 1 1-e 1-e 0+e],[0 0 1 1-e 0+e 0+e],c2);patch([0 0 1 1-e 0+e 0+e],[0 1 1 1-e 1-e 0+e],c3);plot([0 1 1 0 0],[0 0 1 1 0]);disp('b');
  netsum : Sum  Inputs=2  SaturateOnIntegerOverflow=on  InputSameDT=on  OutDataTypeStr=Inherit: Same as first input
      mask Display: e = 0.06;c1 = [120 150 230]/255;c2 = ([120 150 230]+0)/383;c3 = ([120 150 230]+128)/383;patch([0 1 1 0],[0 0 1 1],c1);patch([0 1 1 1-e 1-e 0+e],[0 0 1 1-e 0+e 0+e],c2);patch([0 0 1 1-e 0+e 0+e],[0 1 1 1-e 1-e 0+e],c3);plot([0 1 1 0 0],[0 0 1 1 0]);disp('+');
  purelin : neural/Transfer Functions/purelin
      settings: SourceProductName=Deep Learning Toolbox
  "a{7}" : Outport  InitialOutput=[0;0;0;0;0]
      mask Display: e = 0.06;c1 = [120 230 180]/255;c2 = ([120 230 180]+0)/(256+128);c3 = ([120 230 180]+128)/(256+128);patch([0 1 1 0],[0 0 1 1],c1);patch([0 1 1 1-e 1-e 0+e],[0 0 1 1-e 0+e 0+e],c2);patch([0 0 1 1-e 0+e 0+e],[0 1 1 1-e 1-e 0+e],c3);plot([0 1 1 0 0],[0 0 1 1 0]);
  wires:
  purelin/1 -> "a{7}"/1
  netsum/1 -> purelin/1
  "b{7}"/1 -> netsum/2
  "LW{7,6}"/1("lz{7,6}") -> netsum/1
  Delays 1/1("pd{7,6}") -> "LW{7,6}"/1("ad{7,6}")
  "a{6} "/1 -> Delays 1/1("p{7}")

== system AcasXuClosedLoop_all_comp/networks/Networks_m_7/AcasXu_2_7/Process Input 1 ==
  x : Inport  PortDimensions=5
      mask Display: e = 0.06;c1 = [120 230 180]/255;c2 = ([120 230 180]+0)/(256+128);c3 = ([120 230 180]+128)/(256+128);patch([0 1 1 0],[0 0 1 1],c1);patch([0 1 1 1-e 1-e 0+e],[0 0 1 1-e 0+e 0+e],c2);patch([0 0 1 1-e 0+e 0+e],[0 1 1 1-e 1-e 0+e],c3);plot([0 1 1 0 0],[0 0 1 1 0]);
  p : Outport  PortDimensions=5
      mask Display: e = 0.06;c1 = [120 230 180]/255;c2 = ([120 230 180]+0)/(256+128);c3 = ([120 230 180]+128)/(256+128);patch([0 1 1 0],[0 0 1 1],c1);patch([0 1 1 1-e 1-e 0+e],[0 0 1 1-e 0+e 0+e],c2);patch([0 0 1 1-e 0+e 0+e],[0 1 1 1-e 1-e 0+e],c3);plot([0 1 1 0 0],[0 0 1 1 0]);
  wires:
  x/1 -> p/1

== system AcasXuClosedLoop_all_comp/networks/Networks_m_7/AcasXu_2_7/Process Output 1 ==
  a : Inport  PortDimensions=5
      mask Display: e = 0.06;c1 = [120 230 180]/255;c2 = ([120 230 180]+0)/(256+128);c3 = ([120 230 180]+128)/(256+128);patch([0 1 1 0],[0 0 1 1],c1);patch([0 1 1 1-e 1-e 0+e],[0 0 1 1-e 0+e 0+e],c2);patch([0 0 1 1-e 0+e 0+e],[0 1 1 1-e 1-e 0+e],c3);plot([0 1 1 0 0],[0 0 1 1 0]);
  y : Outport  PortDimensions=5
      mask Display: e = 0.06;c1 = [120 230 180]/255;c2 = ([120 230 180]+0)/(256+128);c3 = ([120 230 180]+128)/(256+128);patch([0 1 1 0],[0 0 1 1],c1);patch([0 1 1 1-e 1-e 0+e],[0 0 1 1-e 0+e 0+e],c2);patch([0 0 1 1-e 0+e 0+e],[0 1 1 1-e 1-e 0+e],c3);plot([0 1 1 0 0],[0 0 1 1 0]);
  wires:
  a/1 -> y/1

== system AcasXuClosedLoop_all_comp/networks/Networks_m_7/AcasXu_3_7/Layer 1 ==
  "p{1}" : Inport  PortDimensions=5
      mask Display: e = 0.06;c1 = [120 230 180]/255;c2 = ([120 230 180]+0)/(256+128);c3 = ([120 230 180]+128)/(256+128);patch([0 1 1 0],[0 0 1 1],c1);patch([0 1 1 1-e 1-e 0+e],[0 0 1 1-e 0+e 0+e],c2);patch([0 0 1 1-e 0+e 0+e],[0 1 1 1-e 1-e 0+e],c3);plot([0 1 1 0 0],[0 0 1 1 0]);
  Delays 1 : Subsystem
      mask Display: e = 0.06;c1 = [120 150 230]/255;c2 = ([120 150 230]+0)/383;c3 = ([120 150 230]+128)/383;patch([0 1 1 0],[0 0 1 1],c1);patch([0 1 1 1-e 1-e 0+e],[0 0 1 1-e 0+e 0+e],c2);patch([0 0 1 1-e 0+e 0+e],[0 1 1 1-e 1-e 0+e],c3);plot([0 1 1 0 0],[0 0 1 1 0]);disp('0');
  "IW{1,1}" : Subsystem
      mask Display: e = 0.06;c1 = [120 150 230]/255;c2 = ([120 150 230]+0)/383;c3 = ([120 150 230]+128)/383;patch([0 1 1 0],[0 0 1 1],c1);patch([0 1 1 1-e 1-e 0+e],[0 0 1 1-e 0+e 0+e],c2);patch([0 0 1 1-e 0+e 0+e],[0 1 1 1-e 1-e 0+e],c3);plot([0 1 1 0 0],[0 0 1 1 0]);disp('W');
  "b{1}" : Constant  Value=[0.043127199999999997481747726624234928749501705169677734375;-0.040191600000000000936228872205902007408440113067626953125;-0.05854489999999999694235697234034887515008449554443359375;-1.005500000000000060396132539608515799045562744140625;-0.477324000000000026044943979286472313106060028076171875;-0.0209289999999999996871391516606308869086205959320068359375;-0.32310899999999997955768549218191765248775482177734375;0.11467199999999999615596379953785799443721771240234375;-0.2009249999999999924948923535339417867362499237060546875;-0.255761999999999989352517104634898714721202850341796875;-0.1542820000000000024709123636057483963668346405029296875;-0.3700769999999999892992263994528912007808685302734375;-0.020573000000000001008526595569492201320827007293701171875;-0.6185810000000000474784656034898944199085235595703125;-0.2185850000000000015187850976872141472995281219482421875;-0.07914630000000000276116907116374932229518890380859375;0.07691220000000000001083577672034152783453464508056640625;0.10266899999999999637534386920378892682492733001708984375;0.007051739999999999918001147847235188237391412258148193359375;-0.371219000000000021177726239329786039888858795166015625;-0.10356200000000000127631238910907995887100696563720703125;-0.6021579999999999710524889451335184276103973388671875;-0.0181814999999999997448707489411390270106494426727294921875;-0.7072950000000000070343730840249918401241302490234375;0.0436355999999999966121322358958423137664794921875;-0.0939765999999999934733097006755997426807880401611328125;-0.3456960000000000032827074392116628587245941162109375;0.122867000000000003989697461292962543666362762451171875;0.115288000000000001588063014423823915421962738037109375;0.4419910000000000227515783990384079515933990478515625;0.042487400000000001554223416633249144069850444793701171875;0.11382799999999999862598798472390626557171344757080078125;0.093844600000000000239452901951153762638568878173828125;-0.219264999999999987689847102956264279782772064208984375;-0.045355499999999999982680520815847557969391345977783203125;0.11269600000000000450395276629933505319058895111083984375;-0.313249999999999972910558199146180413663387298583984375;0.047737200000000000466027216816655709408223628997802734375;-0.304823000000000010611955758577096275985240936279296875;-0.467415999999999998149036173344939015805721282958984375;-0.413897000000000014896528455210500396788120269775390625;0.00173897000000000005563716154455278228851966559886932373046875;0.0511981999999999992656540825919364579021930694580078125;0.1512610000000000065600858079051249660551548004150390625;-0.0639234999999999942144057740733842365443706512451171875;0.0023746700000000001661015769371942951693199574947357177734375;0.10727799999999999835953445881386869587004184722900390625;0.180653000000000008018474773052730597555637359619140625;-0.1558589999999999975432984911094536073505878448486328125;-0.0193119999999999993056665203994270996190607547760009765625]
      mask Display: e = 0.06;c1 = [120 150 230]/255;c2 = ([120 150 230]+0)/383;c3 = ([120 150 230]+128)/383;patch([0 1 1 0],[0 0 1 1],c1);patch([0 1 1 1-e 1-e 0+e],[0 0 1 1-e 0+e 0+e],c2);patch([0 0 1 1-e 0+e 0+e],[0 1 1 1-e 1-e 0+e],c3);plot([0 1 1 0 0],[0 0 1 1 0]);disp('b');
  netsum : Sum  Inputs=2  SaturateOnIntegerOverflow=on  InputSameDT=on  OutDataTypeStr=Inherit: Same as first input
      mask Display: e = 0.06;c1 = [120 150 230]/255;c2 = ([120 150 230]+0)/383;c3 = ([120 150 230]+128)/383;patch([0 1 1 0],[0 0 1 1],c1);patch([0 1 1 1-e 1-e 0+e],[0 0 1 1-e 0+e 0+e],c2);patch([0 0 1 1-e 0+e 0+e],[0 1 1 1-e 1-e 0+e],c3);plot([0 1 1 0 0],[0 0 1 1 0]);disp('+');
  poslin : neural/Transfer Functions/poslin
      settings: SourceProductName=Deep Learning Toolbox  SourceProductBaseCode=NN
  "a{1}" : Outport  InitialOutput=[0;0;0;0;0;0;0;0;0;0;0;0;0;0;0;0;0;0;0;0;0;0;0;0;0;0;0;0;0;0;0;0;0;0;0;0;0;0;0;0;0;0;0;0;0;0;0;0;0;0]
      mask Display: e = 0.06;c1 = [120 230 180]/255;c2 = ([120 230 180]+0)/(256+128);c3 = ([120 230 180]+128)/(256+128);patch([0 1 1 0],[0 0 1 1],c1);patch([0 1 1 1-e 1-e 0+e],[0 0 1 1-e 0+e 0+e],c2);patch([0 0 1 1-e 0+e 0+e],[0 1 1 1-e 1-e 0+e],c3);plot([0 1 1 0 0],[0 0 1 1 0]);
  wires:
  poslin/1 -> "a{1}"/1
  netsum/1 -> poslin/1
  "b{1}"/1 -> netsum/2
  "IW{1,1}"/1("iz{1,1}") -> netsum/1
  Delays 1/1("pd{1,1}") -> "IW{1,1}"/1("pd{1,1}")
  "p{1}"/1 -> Delays 1/1("p{1}")

== system AcasXuClosedLoop_all_comp/networks/Networks_m_7/AcasXu_3_7/Layer 2 ==
  "a{1} " : Inport  PortDimensions=50
      mask Display: e = 0.06;c1 = [120 230 180]/255;c2 = ([120 230 180]+0)/(256+128);c3 = ([120 230 180]+128)/(256+128);patch([0 1 1 0],[0 0 1 1],c1);patch([0 1 1 1-e 1-e 0+e],[0 0 1 1-e 0+e 0+e],c2);patch([0 0 1 1-e 0+e 0+e],[0 1 1 1-e 1-e 0+e],c3);plot([0 1 1 0 0],[0 0 1 1 0]);
  Delays 1 : Subsystem
      mask Display: e = 0.06;c1 = [120 150 230]/255;c2 = ([120 150 230]+0)/383;c3 = ([120 150 230]+128)/383;patch([0 1 1 0],[0 0 1 1],c1);patch([0 1 1 1-e 1-e 0+e],[0 0 1 1-e 0+e 0+e],c2);patch([0 0 1 1-e 0+e 0+e],[0 1 1 1-e 1-e 0+e],c3);plot([0 1 1 0 0],[0 0 1 1 0]);disp('0');
  "LW{2,1}" : Subsystem
      mask Display: e = 0.06;c1 = [120 150 230]/255;c2 = ([120 150 230]+0)/383;c3 = ([120 150 230]+128)/383;patch([0 1 1 0],[0 0 1 1],c1);patch([0 1 1 1-e 1-e 0+e],[0 0 1 1-e 0+e 0+e],c2);patch([0 0 1 1-e 0+e 0+e],[0 1 1 1-e 1-e 0+e],c3);plot([0 1 1 0 0],[0 0 1 1 0]);disp('W');
  "b{2}" : Constant  Value=[-0.2076900000000000134914301952449022792279720306396484375;-0.01088320000000000085937923444134867168031632900238037109375;0.0229485000000000001485478406948459451086819171905517578125;1.0051200000000000134292577058658935129642486572265625;-0.81331600000000003891642563758068718016147613525390625;-0.0168150999999999994916066725636483170092105865478515625;0.0737147999999999969933384136311360634863376617431640625;-0.014960700000000000386979337463344563730061054229736328125;-0.0112388999999999995849098155531464726664125919342041015625;0.7653330000000000410409484175033867359161376953125;0.16039300000000000778754838393069803714752197265625;0.652109999999999967457142702187411487102508544921875;-0.28345900000000001650590775170712731778621673583984375;0.38588400000000000478195261166547425091266632080078125;1.176719999999999988204990586382336914539337158203125;-0.84165100000000003799272008109255693852901458740234375;-0.0088519500000000007899902954022763879038393497467041015625;-0.350854999999999972448705420902115292847156524658203125;0.3924119999999999830464503247640095651149749755859375;-0.67757699999999998485833430095226503908634185791015625;-0.28076299999999998480149088209145702421665191650390625;-0.031511299999999999033040154472473659552633762359619140625;-0.40652100000000002122391151715419255197048187255859375;-0.28266599999999997283595121189136989414691925048828125;0.05258659999999999723474530810563010163605213165283203125;-1.3972700000000000120081722343456931412220001220703125;0.289389999999999980584419745355262421071529388427734375;-0.174217000000000010739853451013914309442043304443359375;0.19910200000000000120081722343456931412220001220703125;-1.0160199999999999231903302643331699073314666748046875;-0.0172245000000000002271516308383070281706750392913818359375;-0.9033130000000000325854898619581945240497589111328125;-0.424673999999999995935695551452226936817169189453125;-0.006565399999999999881838963489144589402712881565093994140625;-0.0148427999999999997438049348374988767318427562713623046875;-0.3300739999999999785273985253297723829746246337890625;0.51564500000000002000177801164682023227214813232421875;-0.010306900000000000783284548333540442399680614471435546875;0.7800409999999999843822706679929979145526885986328125;-0.455793999999999976946440938263549469411373138427734375;-0.0150816999999999999670929895501103601418435573577880859375;0.764782999999999990592414178536273539066314697265625;-0.178887999999999991462829029842396266758441925048828125;-0.40799499999999999655386773156351409852504730224609375;0.65919000000000005368150368667556904256343841552734375;-0.65381199999999994876276332433917559683322906494140625;-0.226833000000000006846079259048565290868282318115234375;-0.58391599999999999059951960589387454092502593994140625;0.96218700000000001448796638214844278991222381591796875;0.473837000000000008181899602277553640305995941162109375]
      mask Display: e = 0.06;c1 = [120 150 230]/255;c2 = ([120 150 230]+0)/383;c3 = ([120 150 230]+128)/383;patch([0 1 1 0],[0 0 1 1],c1);patch([0 1 1 1-e 1-e 0+e],[0 0 1 1-e 0+e 0+e],c2);patch([0 0 1 1-e 0+e 0+e],[0 1 1 1-e 1-e 0+e],c3);plot([0 1 1 0 0],[0 0 1 1 0]);disp('b');
  netsum : Sum  Inputs=2  SaturateOnIntegerOverflow=on  InputSameDT=on  OutDataTypeStr=Inherit: Same as first input
      mask Display: e = 0.06;c1 = [120 150 230]/255;c2 = ([120 150 230]+0)/383;c3 = ([120 150 230]+128)/383;patch([0 1 1 0],[0 0 1 1],c1);patch([0 1 1 1-e 1-e 0+e],[0 0 1 1-e 0+e 0+e],c2);patch([0 0 1 1-e 0+e 0+e],[0 1 1 1-e 1-e 0+e],c3);plot([0 1 1 0 0],[0 0 1 1 0]);disp('+');
  poslin : neural/Transfer Functions/poslin
      settings: SourceProductName=Deep Learning Toolbox  SourceProductBaseCode=NN
  "a{2}" : Outport  InitialOutput=[0;0;0;0;0;0;0;0;0;0;0;0;0;0;0;0;0;0;0;0;0;0;0;0;0;0;0;0;0;0;0;0;0;0;0;0;0;0;0;0;0;0;0;0;0;0;0;0;0;0]
      mask Display: e = 0.06;c1 = [120 230 180]/255;c2 = ([120 230 180]+0)/(256+128);c3 = ([120 230 180]+128)/(256+128);patch([0 1 1 0],[0 0 1 1],c1);patch([0 1 1 1-e 1-e 0+e],[0 0 1 1-e 0+e 0+e],c2);patch([0 0 1 1-e 0+e 0+e],[0 1 1 1-e 1-e 0+e],c3);plot([0 1 1 0 0],[0 0 1 1 0]);
  wires:
  poslin/1 -> "a{2}"/1
  netsum/1 -> poslin/1
  "b{2}"/1 -> netsum/2
  "LW{2,1}"/1("lz{2,1}") -> netsum/1
  Delays 1/1("pd{2,1}") -> "LW{2,1}"/1("ad{2,1}")
  "a{1} "/1 -> Delays 1/1("p{2}")
